simulink model "fft_test_BG"  (Simulink 7.4)
settings: Solver=ode45  StartTime=0.0  StopTime=5000  RelTol=1e-3
libraries: xbsIndex_r4 x4277, casper_library_misc x172, xbsReplacements_r4 x47, casper_library_delays x21, casper_library_reorder x4, casper_library_ffts_twiddle x2, simulink x2, xps_library x1
(part 23 of 37)

== system fft_test_BG/fft_BG_xblock/fft_biplex2/biplex_core/fft_stage_8/sync_delay ==
  In : Inport
  Constant : xbsIndex_r4/Constant  arith_type=Unsigned  const=1  n_bits=2  bin_pt=0  explicit_period=on  period=1  equ=P=C  opselect=C  inp2=PCIN>>17  opr=+  inp1=P  carry=CIN  dbl_ovrd=off  block_type=constant
      settings: block_version=11.4
  Constant1 : xbsIndex_r4/Constant  arith_type=Unsigned  const=0  n_bits=2  bin_pt=0  explicit_period=on  period=1  equ=P=C  opselect=C  inp2=PCIN>>17  opr=+  inp1=P  carry=CIN  dbl_ovrd=off  block_type=constant
      settings: block_version=11.4
  Constant2 : xbsIndex_r4/Constant  arith_type=Unsigned  const=1  n_bits=2  bin_pt=0  explicit_period=on  period=1  equ=P=C  opselect=C  inp2=PCIN>>17  opr=+  inp1=P  carry=CIN  dbl_ovrd=off  block_type=constant
      settings: block_version=11.4
  Constant3 : xbsIndex_r4/Constant  arith_type=Unsigned  const=1  n_bits=1  bin_pt=0  explicit_period=on  period=1  equ=P=C  opselect=C  inp2=PCIN>>17  opr=+  inp1=P  carry=CIN  dbl_ovrd=off  block_type=constant
      settings: block_version=11.4
  Counter : xbsIndex_r4/Counter  cnt_type=Free Running  cnt_to=Inf  operation=Down  start_count=0  cnt_by_val=1  arith_type=Unsigned  n_bits=2  bin_pt=0  load_pin=on  rst=off  en=on  explicit_period=on  period=1  dbl_ovrd=off  use_behavioral_HDL=off  implementation=Fabric  use_rpm=on  block_type=counter
      settings: block_version=11.4
  Logical : xbsIndex_r4/Logical  logical_function=OR  inputs=2  en=off  latency=0  precision=Full  arith_type=Unsigned  n_bits=8  bin_pt=2  align_bp=on  dbl_ovrd=off  block_type=logical
      settings: block_version=11.4
  Mux : xbsIndex_r4/Mux  inputs=2  en=off  latency=0  precision=Full  arith_type="Signed  (2's comp)"  n_bits=8  bin_pt=2  quantization=Truncate  overflow=Wrap  dbl_ovrd=off  block_type=mux
      settings: block_version=11.4
  Relational : xbsIndex_r4/Relational  mode=a=b  en=off  latency=0  dbl_ovrd=off  block_type=relational
  Relational1 : xbsIndex_r4/Relational  mode=a!=b  en=off  latency=0  dbl_ovrd=off  block_type=relational
  warn period Logical0 : xbsReplacements_r4/Warning  SystemSampleTime=-1  period=1
  warn period Relational10 : xbsReplacements_r4/Warning  SystemSampleTime=-1  period=1
  Out : Outport
  wires:
  In/1 -> Mux/2, Logical/1, Counter/1
  Counter/1 -> Relational1/1, Relational/2
  Relational1/1 -> Logical/2
  Constant1/1 -> Relational1/2
  Relational/1 -> Mux/3
  Constant/1 -> Relational/1
  Mux/1 -> Out/1
  Constant3/1 -> Mux/1
  Logical/1 -> Counter/3
  Constant2/1 -> Counter/2

== system fft_test_BG/fft_BG_xblock/fft_biplex2/biplex_cplx_unscrambler/reorder/sync_delay_en ==
  In : Inport
  en : Inport  Port=2
  Constant : xbsIndex_r4/Constant  arith_type=Unsigned  const=1  n_bits=9  bin_pt=0  explicit_period=on  period=1  equ=P=C  opselect=C  inp2=PCIN>>17  opr=+  inp1=P  carry=CIN  dbl_ovrd=off  block_type=constant
      settings: block_version=11.4
  Constant1 : xbsIndex_r4/Constant  arith_type=Unsigned  const=0  n_bits=9  bin_pt=0  explicit_period=on  period=1  equ=P=C  opselect=C  inp2=PCIN>>17  opr=+  inp1=P  carry=CIN  dbl_ovrd=off  block_type=constant
      settings: block_version=11.4
  Constant2 : xbsIndex_r4/Constant  arith_type=Unsigned  const=256  n_bits=9  bin_pt=0  explicit_period=on  period=1  equ=P=C  opselect=C  inp2=PCIN>>17  opr=+  inp1=P  carry=CIN  dbl_ovrd=off  block_type=constant
      settings: block_version=11.4
  Constant3 : xbsIndex_r4/Constant  arith_type=Unsigned  const=1  n_bits=1  bin_pt=0  explicit_period=on  period=1  equ=P=C  opselect=C  inp2=PCIN>>17  opr=+  inp1=P  carry=CIN  dbl_ovrd=off  block_type=constant
      settings: block_version=11.4
  Counter : xbsIndex_r4/Counter  cnt_type=Free Running  cnt_to=Inf  operation=Down  start_count=0  cnt_by_val=1  arith_type=Unsigned  n_bits=9  bin_pt=0  load_pin=on  rst=off  en=on  explicit_period=on  period=1  dbl_ovrd=off  use_behavioral_HDL=off  implementation=Fabric  use_rpm=on  block_type=counter
      settings: block_version=11.4
  Logical : xbsIndex_r4/Logical  logical_function=OR  inputs=2  en=off  latency=0  precision=Full  arith_type=Unsigned  n_bits=8  bin_pt=2  align_bp=on  dbl_ovrd=off  block_type=logical
      settings: block_version=11.4
  Logical1 : xbsIndex_r4/Logical  logical_function=AND  inputs=2  en=off  latency=0  precision=Full  arith_type=Unsigned  n_bits=8  bin_pt=2  align_bp=on  dbl_ovrd=off  block_type=logical
      settings: block_version=11.4
  Mux : xbsIndex_r4/Mux  inputs=2  en=off  latency=0  precision=Full  arith_type="Signed  (2's comp)"  n_bits=8  bin_pt=2  quantization=Truncate  overflow=Wrap  dbl_ovrd=off  block_type=mux
      settings: block_version=11.4
  Relational : xbsIndex_r4/Relational  mode=a=b  en=off  latency=0  dbl_ovrd=off  block_type=relational
  Relational1 : xbsIndex_r4/Relational  mode=a!=b  en=off  latency=0  dbl_ovrd=off  block_type=relational
  warn period Logical0 : xbsReplacements_r4/Warning  SystemSampleTime=-1  period=1
  warn period Logical10 : xbsReplacements_r4/Warning  SystemSampleTime=-1  period=1
  warn period Relational10 : xbsReplacements_r4/Warning  SystemSampleTime=-1  period=1
  Out : Outport
  wires:
  In/1 -> Mux/2, Logical/1, Counter/1
  Counter/1 -> Relational1/1, Relational/2
  Relational1/1 -> Logical/2
  Constant1/1 -> Relational1/2
  Relational/1 -> Mux/3
  Constant/1 -> Relational/1
  Mux/1 -> Out/1
  Constant3/1 -> Mux/1
  Logical1/1 -> Counter/3
  en/1 -> Logical1/2
  Logical/1 -> Logical1/1
  Constant2/1 -> Counter/2

== system fft_test_BG/fft_BG_xblock/fft_biplex2/biplex_cplx_unscrambler/reorder1/sync_delay_en ==
  In : Inport
  en : Inport  Port=2
  Constant : xbsIndex_r4/Constant  arith_type=Unsigned  const=1  n_bits=9  bin_pt=0  explicit_period=on  period=1  equ=P=C  opselect=C  inp2=PCIN>>17  opr=+  inp1=P  carry=CIN  dbl_ovrd=off  block_type=constant
      settings: block_version=11.4
  Constant1 : xbsIndex_r4/Constant  arith_type=Unsigned  const=0  n_bits=9  bin_pt=0  explicit_period=on  period=1  equ=P=C  opselect=C  inp2=PCIN>>17  opr=+  inp1=P  carry=CIN  dbl_ovrd=off  block_type=constant
      settings: block_version=11.4
  Constant2 : xbsIndex_r4/Constant  arith_type=Unsigned  const=256  n_bits=9  bin_pt=0  explicit_period=on  period=1  equ=P=C  opselect=C  inp2=PCIN>>17  opr=+  inp1=P  carry=CIN  dbl_ovrd=off  block_type=constant
      settings: block_version=11.4
  Constant3 : xbsIndex_r4/Constant  arith_type=Unsigned  const=1  n_bits=1  bin_pt=0  explicit_period=on  period=1  equ=P=C  opselect=C  inp2=PCIN>>17  opr=+  inp1=P  carry=CIN  dbl_ovrd=off  block_type=constant
      settings: block_version=11.4
  Counter : xbsIndex_r4/Counter  cnt_type=Free Running  cnt_to=Inf  operation=Down  start_count=0  cnt_by_val=1  arith_type=Unsigned  n_bits=9  bin_pt=0  load_pin=on  rst=off  en=on  explicit_period=on  period=1  dbl_ovrd=off  use_behavioral_HDL=off  implementation=Fabric  use_rpm=on  block_type=counter
      settings: block_version=11.4
  Logical : xbsIndex_r4/Logical  logical_function=OR  inputs=2  en=off  latency=0  precision=Full  arith_type=Unsigned  n_bits=8  bin_pt=2  align_bp=on  dbl_ovrd=off  block_type=logical
      settings: block_version=11.4
  Logical1 : xbsIndex_r4/Logical  logical_function=AND  inputs=2  en=off  latency=0  precision=Full  arith_type=Unsigned  n_bits=8  bin_pt=2  align_bp=on  dbl_ovrd=off  block_type=logical
      settings: block_version=11.4
  Mux : xbsIndex_r4/Mux  inputs=2  en=off  latency=0  precision=Full  arith_type="Signed  (2's comp)"  n_bits=8  bin_pt=2  quantization=Truncate  overflow=Wrap  dbl_ovrd=off  block_type=mux
      settings: block_version=11.4
  Relational : xbsIndex_r4/Relational  mode=a=b  en=off  latency=0  dbl_ovrd=off  block_type=relational
  Relational1 : xbsIndex_r4/Relational  mode=a!=b  en=off  latency=0  dbl_ovrd=off  block_type=relational
  warn period Logical0 : xbsReplacements_r4/Warning  SystemSampleTime=-1  period=1
  warn period Logical10 : xbsReplacements_r4/Warning  SystemSampleTime=-1  period=1
  warn period Relational10 : xbsReplacements_r4/Warning  SystemSampleTime=-1  period=1
  Out : Outport
  wires:
  In/1 -> Mux/2, Logical/1, Counter/1
  Counter/1 -> Relational1/1, Relational/2
  Relational1/1 -> Logical/2
  Constant1/1 -> Relational1/2
  Relational/1 -> Mux/3
  Constant/1 -> Relational/1
  Mux/1 -> Out/1
  Constant3/1 -> Mux/1
  Logical1/1 -> Counter/3
  en/1 -> Logical1/2
  Logical/1 -> Logical1/1
  Constant2/1 -> Counter/2

== system fft_test_BG/fft_BG_xblock/fft_direct_xblock/butterfly1_0/twiddle/c_to_ri_a ==
  c : Inport
  force_im : xbsIndex_r4/Reinterpret  force_arith_type=on  arith_type="Signed  (2's comp)"  force_bin_pt=on  bin_pt=16  block_type=reinterpret
      settings: infoedit="Changes signal type without altering the binary representation.   You can changed the signal between signed and unsigned, and relocate the binary point.<br><br>Hardware notes: In hardware this block costs nothing.<br><br>Example:  Suppose the input is 6 bits wide, signed, with 2 fractional bits, and the output is forced to unsigned with 0 fractional bits.  Then an input of -2.0 (1110.00 in binary 2's complement) becomes an output of 56 (111000 in binary)."
  force_re : xbsIndex_r4/Reinterpret  force_arith_type=on  arith_type="Signed  (2's comp)"  force_bin_pt=on  bin_pt=16  block_type=reinterpret
      settings: infoedit="Changes signal type without altering the binary representation.   You can changed the signal between signed and unsigned, and relocate the binary point.<br><br>Hardware notes: In hardware this block costs nothing.<br><br>Example:  Suppose the input is 6 bits wide, signed, with 2 fractional bits, and the output is forced to unsigned with 0 fractional bits.  Then an input of -2.0 (1110.00 in binary 2's complement) becomes an output of 56 (111000 in binary)."
  slice_im : xbsIndex_r4/Slice  nbits=17  boolean_output=off  mode=Lower Bit Location + Width  bit1=0  base1=MSB of Input  bit0=0  base0=LSB of Input  dbl_ovrd=off  block_type=slice
  slice_re : xbsIndex_r4/Slice  nbits=17  boolean_output=off  mode=Upper Bit Location + Width  bit1=0  base1=MSB of Input  bit0=0  base0=LSB of Input  dbl_ovrd=off  block_type=slice
  re : Outport
  im : Outport  Port=2
  wires:
  c/1 -> slice_re/1, slice_im/1
  slice_re/1 -> force_re/1
  slice_im/1 -> force_im/1
  force_re/1 -> re/1
  force_im/1 -> im/1

== system fft_test_BG/fft_BG_xblock/fft_direct_xblock/butterfly1_0/twiddle/c_to_ri_b ==
  c : Inport
  force_im : xbsIndex_r4/Reinterpret  force_arith_type=on  arith_type="Signed  (2's comp)"  force_bin_pt=on  bin_pt=16  block_type=reinterpret
      settings: infoedit="Changes signal type without altering the binary representation.   You can changed the signal between signed and unsigned, and relocate the binary point.<br><br>Hardware notes: In hardware this block costs nothing.<br><br>Example:  Suppose the input is 6 bits wide, signed, with 2 fractional bits, and the output is forced to unsigned with 0 fractional bits.  Then an input of -2.0 (1110.00 in binary 2's complement) becomes an output of 56 (111000 in binary)."
  force_re : xbsIndex_r4/Reinterpret  force_arith_type=on  arith_type="Signed  (2's comp)"  force_bin_pt=on  bin_pt=16  block_type=reinterpret
      settings: infoedit="Changes signal type without altering the binary representation.   You can changed the signal between signed and unsigned, and relocate the binary point.<br><br>Hardware notes: In hardware this block costs nothing.<br><br>Example:  Suppose the input is 6 bits wide, signed, with 2 fractional bits, and the output is forced to unsigned with 0 fractional bits.  Then an input of -2.0 (1110.00 in binary 2's complement) becomes an output of 56 (111000 in binary)."
  slice_im : xbsIndex_r4/Slice  nbits=17  boolean_output=off  mode=Lower Bit Location + Width  bit1=0  base1=MSB of Input  bit0=0  base0=LSB of Input  dbl_ovrd=off  block_type=slice
  slice_re : xbsIndex_r4/Slice  nbits=17  boolean_output=off  mode=Upper Bit Location + Width  bit1=0  base1=MSB of Input  bit0=0  base0=LSB of Input  dbl_ovrd=off  block_type=slice
  re : Outport
  im : Outport  Port=2
  wires:
  c/1 -> slice_re/1, slice_im/1
  slice_re/1 -> force_re/1
  slice_im/1 -> force_im/1
  force_re/1 -> re/1
  force_im/1 -> im/1

== system fft_test_BG/fft_BG_xblock/fft_direct_xblock/butterfly1_0/twiddle/c_to_ri_w ==
  c : Inport
  force_im : xbsIndex_r4/Reinterpret  force_arith_type=on  arith_type="Signed  (2's comp)"  force_bin_pt=on  bin_pt=15  block_type=reinterpret
      settings: infoedit="Changes signal type without altering the binary representation.   You can changed the signal between signed and unsigned, and relocate the binary point.<br><br>Hardware notes: In hardware this block costs nothing.<br><br>Example:  Suppose the input is 6 bits wide, signed, with 2 fractional bits, and the output is forced to unsigned with 0 fractional bits.  Then an input of -2.0 (1110.00 in binary 2's complement) becomes an output of 56 (111000 in binary)."
  force_re : xbsIndex_r4/Reinterpret  force_arith_type=on  arith_type="Signed  (2's comp)"  force_bin_pt=on  bin_pt=15  block_type=reinterpret
      settings: infoedit="Changes signal type without altering the binary representation.   You can changed the signal between signed and unsigned, and relocate the binary point.<br><br>Hardware notes: In hardware this block costs nothing.<br><br>Example:  Suppose the input is 6 bits wide, signed, with 2 fractional bits, and the output is forced to unsigned with 0 fractional bits.  Then an input of -2.0 (1110.00 in binary 2's complement) becomes an output of 56 (111000 in binary)."
  slice_im : xbsIndex_r4/Slice  nbits=17  boolean_output=off  mode=Lower Bit Location + Width  bit1=0  base1=MSB of Input  bit0=0  base0=LSB of Input  dbl_ovrd=off  block_type=slice
  slice_re : xbsIndex_r4/Slice  nbits=17  boolean_output=off  mode=Upper Bit Location + Width  bit1=0  base1=MSB of Input  bit0=0  base0=LSB of Input  dbl_ovrd=off  block_type=slice
  re : Outport
  im : Outport  Port=2
  wires:
  c/1 -> slice_re/1, slice_im/1
  slice_re/1 -> force_re/1
  slice_im/1 -> force_im/1
  force_re/1 -> re/1
  force_im/1 -> im/1

== system fft_test_BG/fft_BG_xblock/fft_direct_xblock/butterfly1_0/twiddle/coeff_gen ==
  rst : Inport
  Counter : xbsIndex_r4/Counter  cnt_type=Free Running  cnt_to=Inf  operation=Up  start_count=0  cnt_by_val=1  arith_type=Unsigned  n_bits=8  bin_pt=0  load_pin=off  rst=on  en=off  explicit_period=on  period=1  dbl_ovrd=off  use_behavioral_HDL=off  implementation=Fabric  block_type=counter
      settings: block_version=11.4
  ROM : xbsIndex_r4/ROM  depth=256  initVector=[1 0.99993896484375 0.99969482421875 0.99932861328125 0.998809814453125 0.99810791015625 0.997283935546875 0.996307373046875 0.99517822265625 0.993896484375 0.99249267578125 0.99090576171875 0.989166259765625 0.9873046875 0.98529052734375 0.98309326171875 0.98077392578125 0.97833251953125 0.9757080078125 0.972930908203125 0.97003173828125 0.96697998046875 0.963775634765625 0.960418701171875 0.956939697265625 0.95330810546875 0.94952392578125 0.94561767578125 0.941558837890625 0.937347412109375 0.9329833984375 0.928497314453125 0.92388916015625 0.91912841796875 0.914215087890625 0.9091796875 0.90399169921875 0.898681640625 0.893218994140625 0.88763427734375 0.881927490234375 0.876068115234375 0.870086669921875 0.863983154296875 0.85772705078125 0.851348876953125 0.8448486328125 0.838226318359375 0.83148193359375 0.8245849609375 0.817596435546875 0.810455322265625 0.80322265625 0.79583740234375 0.788360595703125 0.780731201171875 0.77301025390625 0.765167236328125 0.7572021484375 0.7491455078125 0.740936279296875 0.732666015625 0.7242431640625 0.715728759765625 0.70709228515625 0.6983642578125 0.689544677734375 0.68060302734375 0.67156982421875 0.66241455078125 0.653167724609375 0.643829345703125 0.6343994140625 0.624847412109375 0.615234375 0.605499267578125 0.595703125 0.585784912109375 0.5758056640625 0.56573486328125 0.555572509765625 0.545318603515625 0.535003662109375 0.52459716796875 0.51409912109375 0.5035400390625 0.492889404296875 0.482177734375 0.471405029296875 0.460540771484375 0.449615478515625 0.438629150390625 0.42755126953125 0.41644287109375 0.405242919921875 0.39398193359375 0.3826904296875 0.371307373046875 0.359893798828125 0.348419189453125 0.336883544921875 0.3253173828125 0.313690185546875 0.302001953125 0.290283203125 0.278533935546875 0.2667236328125 0.254852294921875 0.24298095703125 0.231048583984375 0.2191162109375 0.207122802734375 0.195098876953125 0.18304443359375 0.17095947265625 0.158843994140625 0.146728515625 0.13458251953125 0.122406005859375 0.1102294921875 0.0980224609375 0.085784912109375 0.073577880859375 0.061309814453125 0.049072265625 0.03680419921875 0.0245361328125 0.01226806640625 0 -0.01226806640625 -0.0245361328125 -0.03680419921875 -0.049072265625 -0.061309814453125 -0.073577880859375 -0.085784912109375 -0.0980224609375 -0.1102294921875 -0.122406005859375 -0.13458251953125 -0.146728515625 -0.158843994140625 -0.17095947265625 -0.18304443359375 -0.195098876953125 -0.207122802734375 -0.2191162109375 -0.231048583984375 -0.24298095703125 -0.254852294921875 -0.2667236328125 -0.278533935546875 -0.290283203125 -0.302001953125 -0.313690185546875 -0.3253173828125 -0.336883544921875 -0.348419189453125 -0.359893798828125 -0.371307373046875 -0.3826904296875 -0.39398193359375 -0.405242919921875 -0.41644287109375 -0.42755126953125 -0.438629150390625 -0.449615478515625 -0.460540771484375 -0.471405029296875 -0.482177734375 -0.492889404296875 -0.5035400390625 -0.51409912109375 -0.52459716796875 -0.535003662109375 -0.545318603515625 -0.555572509765625 -0.56573486328125 -0.5758056640625 -0.585784912109375 -0.595703125 -0.605499267578125 -0.615234375 -0.624847412109375 -0.6343994140625 -0.643829345703125 -0.653167724609375 -0.66241455078125 -0.67156982421875 -0.68060302734375 -0.689544677734375 -0.6983642578125 -0.70709228515625 -0.715728759765625 -0.7242431640625 -0.732666015625 -0.740936279296875 -0.7491455078125 -0.7572021484375 -0.765167236328125 -0.77301025390625 -0.780731201171875 -0.788360595703125 -0.79583740234375 -0.80322265625 -0.810455322265625 -0.817596435546875 -0.8245849609375 -0.83148193359375 -0.838226318359375 -0.8448486328125 -0.851348876953125 -0.85772705078125 -0.863983154296875 -0.870086669921875 -0.876068115234375 -0.881927490234375 -0.88763427734375 -0.893218994140625 -0.898681640625 -0.90399169921875 -0.9091796875 -0.914215087890625 -0.91912841796875 -0.92388916015625 -0.928497314453125 -0.9329833984375 -0.937347412109375 -0.941558837890625 -0.94561767578125 ...(+469 chars)]  distributed_mem=Block RAM  rst=off  init_reg=0  en=off  latency=2  arith_type="Signed  (2's comp)"  n_bits=17  bin_pt=15  dbl_ovrd=off  optimize=Area  use_rpm=on  block_type=sprom
      settings: block_version=11.4
  ROM1 : xbsIndex_r4/ROM  depth=256  initVector=[0 -0.01226806640625 -0.0245361328125 -0.03680419921875 -0.049072265625 -0.061309814453125 -0.073577880859375 -0.085784912109375 -0.0980224609375 -0.1102294921875 -0.122406005859375 -0.13458251953125 -0.146728515625 -0.158843994140625 -0.17095947265625 -0.18304443359375 -0.195098876953125 -0.207122802734375 -0.2191162109375 -0.231048583984375 -0.24298095703125 -0.254852294921875 -0.2667236328125 -0.278533935546875 -0.290283203125 -0.302001953125 -0.313690185546875 -0.3253173828125 -0.336883544921875 -0.348419189453125 -0.359893798828125 -0.371307373046875 -0.3826904296875 -0.39398193359375 -0.405242919921875 -0.41644287109375 -0.42755126953125 -0.438629150390625 -0.449615478515625 -0.460540771484375 -0.471405029296875 -0.482177734375 -0.492889404296875 -0.5035400390625 -0.51409912109375 -0.52459716796875 -0.535003662109375 -0.545318603515625 -0.555572509765625 -0.56573486328125 -0.5758056640625 -0.585784912109375 -0.595703125 -0.605499267578125 -0.615234375 -0.624847412109375 -0.6343994140625 -0.643829345703125 -0.653167724609375 -0.66241455078125 -0.67156982421875 -0.68060302734375 -0.689544677734375 -0.6983642578125 -0.70709228515625 -0.715728759765625 -0.7242431640625 -0.732666015625 -0.740936279296875 -0.7491455078125 -0.7572021484375 -0.765167236328125 -0.77301025390625 -0.780731201171875 -0.788360595703125 -0.79583740234375 -0.80322265625 -0.810455322265625 -0.817596435546875 -0.8245849609375 -0.83148193359375 -0.838226318359375 -0.8448486328125 -0.851348876953125 -0.85772705078125 -0.863983154296875 -0.870086669921875 -0.876068115234375 -0.881927490234375 -0.88763427734375 -0.893218994140625 -0.898681640625 -0.90399169921875 -0.9091796875 -0.914215087890625 -0.91912841796875 -0.92388916015625 -0.928497314453125 -0.9329833984375 -0.937347412109375 -0.941558837890625 -0.94561767578125 -0.94952392578125 -0.95330810546875 -0.956939697265625 -0.960418701171875 -0.963775634765625 -0.96697998046875 -0.97003173828125 -0.972930908203125 -0.9757080078125 -0.97833251953125 -0.98077392578125 -0.98309326171875 -0.98529052734375 -0.9873046875 -0.989166259765625 -0.99090576171875 -0.99249267578125 -0.993896484375 -0.99517822265625 -0.996307373046875 -0.997283935546875 -0.99810791015625 -0.998809814453125 -0.99932861328125 -0.99969482421875 -0.99993896484375 -1 -0.99993896484375 -0.99969482421875 -0.99932861328125 -0.998809814453125 -0.99810791015625 -0.997283935546875 -0.996307373046875 -0.99517822265625 -0.993896484375 -0.99249267578125 -0.99090576171875 -0.989166259765625 -0.9873046875 -0.98529052734375 -0.98309326171875 -0.98077392578125 -0.97833251953125 -0.9757080078125 -0.972930908203125 -0.97003173828125 -0.96697998046875 -0.963775634765625 -0.960418701171875 -0.956939697265625 -0.95330810546875 -0.94952392578125 -0.94561767578125 -0.941558837890625 -0.937347412109375 -0.9329833984375 -0.928497314453125 -0.92388916015625 -0.91912841796875 -0.914215087890625 -0.9091796875 -0.90399169921875 -0.898681640625 -0.893218994140625 -0.88763427734375 -0.881927490234375 -0.876068115234375 -0.870086669921875 -0.863983154296875 -0.85772705078125 -0.851348876953125 -0.8448486328125 -0.838226318359375 -0.83148193359375 -0.8245849609375 -0.817596435546875 -0.810455322265625 -0.80322265625 -0.79583740234375 -0.788360595703125 -0.780731201171875 -0.77301025390625 -0.765167236328125 -0.7572021484375 -0.7491455078125 -0.740936279296875 -0.732666015625 -0.7242431640625 -0.715728759765625 -0.70709228515625 -0.6983642578125 -0.689544677734375 -0.68060302734375 -0.67156982421875 -0.66241455078125 -0.653167724609375 -0.643829345703125 -0.6343994140625 -0.624847412109375 -0.615234375 -0.605499267578125 -0.595703125 -0.585784912109375 -0.5758056640625 -0.56573486328125 -0.555572509765625 -0.545318603515625 -0.535003662109375 -0.52459716796875 -0.51409912109375 -0.5035400390625 -0.492889404296875 -0.482177734375 -0.471405029296875 -0.460540771484375 -0.449615478515625 -0.438629150390625 -0.42755126953125 -0.41644287109375 -0.405242919921875 ...(+594 chars)]  distributed_mem=Block RAM  rst=off  init_reg=0  en=off  latency=2  arith_type="Signed  (2's comp)"  n_bits=17  bin_pt=15  dbl_ovrd=off  optimize=Area  use_rpm=on  block_type=sprom
      settings: block_version=11.4
  Slice : xbsIndex_r4/Slice  nbits=8  boolean_output=off  mode=Upper Bit Location + Width  bit1=0  base1=MSB of Input  bit0=0  base0=LSB of Input  dbl_ovrd=off  block_type=slice
  ri_to_c : casper_library_misc/ri_to_c  SystemSampleTime=-1
  w : Outport
  wires:
  Slice/1 -> ROM1/1, ROM/1
  ri_to_c/1 -> w/1
  ROM1/1 -> ri_to_c/2
  ROM/1 -> ri_to_c/1
  Counter/1 -> Slice/1
  rst/1 -> Counter/1

== system fft_test_BG/fft_BG_xblock/fft_direct_xblock/butterfly1_0/twiddle/mults ==
  a1 : Inport
  b1 : Inport  Port=2
  a2 : Inport  Port=3
  b2 : Inport  Port=4
  a3 : Inport  Port=5
  b3 : Inport  Port=6
  a4 : Inport  Port=7
  b4 : Inport  Port=8
  mult_{1..4} (4 alike) : xbsIndex_r4/Mult  precision=Full  arith_type="Signed  (2's comp)"  n_bits=16  bin_pt=14  quantization="Round  (unbiased: +/- Inf)"  overflow=Saturate  en=off  latency=2  dbl_ovrd=off  use_behavioral_HDL=off  opt=Speed  use_embedded=on  optimum_pipeline=off  pipeline=on  use_rpm=on  placement_style=Triangular  block_type=mult
      settings: block_version=11.4
  c1 : Outport
  c2 : Outport  Port=2
  c3 : Outport  Port=3
  c4 : Outport  Port=4
  wires:
  mult_4/1 -> c4/1
  b4/1 -> mult_4/2
  a4/1 -> mult_4/1
  mult_3/1 -> c3/1
  b3/1 -> mult_3/2
  a3/1 -> mult_3/1
  mult_2/1 -> c2/1
  b2/1 -> mult_2/2
  a2/1 -> mult_2/1
  mult_1/1 -> c1/1
  b1/1 -> mult_1/2
  a1/1 -> mult_1/1

== system fft_test_BG/fft_BG_xblock/fft_direct_xblock/butterfly1_1/twiddle/c_to_ri_a ==
  c : Inport
  force_im : xbsIndex_r4/Reinterpret  force_arith_type=on  arith_type="Signed  (2's comp)"  force_bin_pt=on  bin_pt=16  block_type=reinterpret
      settings: infoedit="Changes signal type without altering the binary representation.   You can changed the signal between signed and unsigned, and relocate the binary point.<br><br>Hardware notes: In hardware this block costs nothing.<br><br>Example:  Suppose the input is 6 bits wide, signed, with 2 fractional bits, and the output is forced to unsigned with 0 fractional bits.  Then an input of -2.0 (1110.00 in binary 2's complement) becomes an output of 56 (111000 in binary)."
  force_re : xbsIndex_r4/Reinterpret  force_arith_type=on  arith_type="Signed  (2's comp)"  force_bin_pt=on  bin_pt=16  block_type=reinterpret
      settings: infoedit="Changes signal type without altering the binary representation.   You can changed the signal between signed and unsigned, and relocate the binary point.<br><br>Hardware notes: In hardware this block costs nothing.<br><br>Example:  Suppose the input is 6 bits wide, signed, with 2 fractional bits, and the output is forced to unsigned with 0 fractional bits.  Then an input of -2.0 (1110.00 in binary 2's complement) becomes an output of 56 (111000 in binary)."
  slice_im : xbsIndex_r4/Slice  nbits=17  boolean_output=off  mode=Lower Bit Location + Width  bit1=0  base1=MSB of Input  bit0=0  base0=LSB of Input  dbl_ovrd=off  block_type=slice
  slice_re : xbsIndex_r4/Slice  nbits=17  boolean_output=off  mode=Upper Bit Location + Width  bit1=0  base1=MSB of Input  bit0=0  base0=LSB of Input  dbl_ovrd=off  block_type=slice
  re : Outport
  im : Outport  Port=2
  wires:
  c/1 -> slice_re/1, slice_im/1
  slice_re/1 -> force_re/1
  slice_im/1 -> force_im/1
  force_re/1 -> re/1
  force_im/1 -> im/1

== system fft_test_BG/fft_BG_xblock/fft_direct_xblock/butterfly1_1/twiddle/c_to_ri_b ==
  c : Inport
  force_im : xbsIndex_r4/Reinterpret  force_arith_type=on  arith_type="Signed  (2's comp)"  force_bin_pt=on  bin_pt=16  block_type=reinterpret
      settings: infoedit="Changes signal type without altering the binary representation.   You can changed the signal between signed and unsigned, and relocate the binary point.<br><br>Hardware notes: In hardware this block costs nothing.<br><br>Example:  Suppose the input is 6 bits wide, signed, with 2 fractional bits, and the output is forced to unsigned with 0 fractional bits.  Then an input of -2.0 (1110.00 in binary 2's complement) becomes an output of 56 (111000 in binary)."
  force_re : xbsIndex_r4/Reinterpret  force_arith_type=on  arith_type="Signed  (2's comp)"  force_bin_pt=on  bin_pt=16  block_type=reinterpret
      settings: infoedit="Changes signal type without altering the binary representation.   You can changed the signal between signed and unsigned, and relocate the binary point.<br><br>Hardware notes: In hardware this block costs nothing.<br><br>Example:  Suppose the input is 6 bits wide, signed, with 2 fractional bits, and the output is forced to unsigned with 0 fractional bits.  Then an input of -2.0 (1110.00 in binary 2's complement) becomes an output of 56 (111000 in binary)."
  slice_im : xbsIndex_r4/Slice  nbits=17  boolean_output=off  mode=Lower Bit Location + Width  bit1=0  base1=MSB of Input  bit0=0  base0=LSB of Input  dbl_ovrd=off  block_type=slice
  slice_re : xbsIndex_r4/Slice  nbits=17  boolean_output=off  mode=Upper Bit Location + Width  bit1=0  base1=MSB of Input  bit0=0  base0=LSB of Input  dbl_ovrd=off  block_type=slice
  re : Outport
  im : Outport  Port=2
  wires:
  c/1 -> slice_re/1, slice_im/1
  slice_re/1 -> force_re/1
  slice_im/1 -> force_im/1
  force_re/1 -> re/1
  force_im/1 -> im/1

== system fft_test_BG/fft_BG_xblock/fft_direct_xblock/butterfly1_1/twiddle/c_to_ri_w ==
  c : Inport
  force_im : xbsIndex_r4/Reinterpret  force_arith_type=on  arith_type="Signed  (2's comp)"  force_bin_pt=on  bin_pt=15  block_type=reinterpret
      settings: infoedit="Changes signal type without altering the binary representation.   You can changed the signal between signed and unsigned, and relocate the binary point.<br><br>Hardware notes: In hardware this block costs nothing.<br><br>Example:  Suppose the input is 6 bits wide, signed, with 2 fractional bits, and the output is forced to unsigned with 0 fractional bits.  Then an input of -2.0 (1110.00 in binary 2's complement) becomes an output of 56 (111000 in binary)."
  force_re : xbsIndex_r4/Reinterpret  force_arith_type=on  arith_type="Signed  (2's comp)"  force_bin_pt=on  bin_pt=15  block_type=reinterpret
      settings: infoedit="Changes signal type without altering the binary representation.   You can changed the signal between signed and unsigned, and relocate the binary point.<br><br>Hardware notes: In hardware this block costs nothing.<br><br>Example:  Suppose the input is 6 bits wide, signed, with 2 fractional bits, and the output is forced to unsigned with 0 fractional bits.  Then an input of -2.0 (1110.00 in binary 2's complement) becomes an output of 56 (111000 in binary)."
  slice_im : xbsIndex_r4/Slice  nbits=17  boolean_output=off  mode=Lower Bit Location + Width  bit1=0  base1=MSB of Input  bit0=0  base0=LSB of Input  dbl_ovrd=off  block_type=slice
  slice_re : xbsIndex_r4/Slice  nbits=17  boolean_output=off  mode=Upper Bit Location + Width  bit1=0  base1=MSB of Input  bit0=0  base0=LSB of Input  dbl_ovrd=off  block_type=slice
  re : Outport
  im : Outport  Port=2
  wires:
  c/1 -> slice_re/1, slice_im/1
  slice_re/1 -> force_re/1
  slice_im/1 -> force_im/1
  force_re/1 -> re/1
  force_im/1 -> im/1

== system fft_test_BG/fft_BG_xblock/fft_direct_xblock/butterfly1_1/twiddle/coeff_gen ==
  rst : Inport
  Counter : xbsIndex_r4/Counter  cnt_type=Free Running  cnt_to=Inf  operation=Up  start_count=0  cnt_by_val=1  arith_type=Unsigned  n_bits=8  bin_pt=0  load_pin=off  rst=on  en=off  explicit_period=on  period=1  dbl_ovrd=off  use_behavioral_HDL=off  implementation=Fabric  block_type=counter
      settings: block_version=11.4
  ROM : xbsIndex_r4/ROM  depth=256  initVector=[1 0.99993896484375 0.99969482421875 0.99932861328125 0.998809814453125 0.99810791015625 0.997283935546875 0.996307373046875 0.99517822265625 0.993896484375 0.99249267578125 0.99090576171875 0.989166259765625 0.9873046875 0.98529052734375 0.98309326171875 0.98077392578125 0.97833251953125 0.9757080078125 0.972930908203125 0.97003173828125 0.96697998046875 0.963775634765625 0.960418701171875 0.956939697265625 0.95330810546875 0.94952392578125 0.94561767578125 0.941558837890625 0.937347412109375 0.9329833984375 0.928497314453125 0.92388916015625 0.91912841796875 0.914215087890625 0.9091796875 0.90399169921875 0.898681640625 0.893218994140625 0.88763427734375 0.881927490234375 0.876068115234375 0.870086669921875 0.863983154296875 0.85772705078125 0.851348876953125 0.8448486328125 0.838226318359375 0.83148193359375 0.8245849609375 0.817596435546875 0.810455322265625 0.80322265625 0.79583740234375 0.788360595703125 0.780731201171875 0.77301025390625 0.765167236328125 0.7572021484375 0.7491455078125 0.740936279296875 0.732666015625 0.7242431640625 0.715728759765625 0.70709228515625 0.6983642578125 0.689544677734375 0.68060302734375 0.67156982421875 0.66241455078125 0.653167724609375 0.643829345703125 0.6343994140625 0.624847412109375 0.615234375 0.605499267578125 0.595703125 0.585784912109375 0.5758056640625 0.56573486328125 0.555572509765625 0.545318603515625 0.535003662109375 0.52459716796875 0.51409912109375 0.5035400390625 0.492889404296875 0.482177734375 0.471405029296875 0.460540771484375 0.449615478515625 0.438629150390625 0.42755126953125 0.41644287109375 0.405242919921875 0.39398193359375 0.3826904296875 0.371307373046875 0.359893798828125 0.348419189453125 0.336883544921875 0.3253173828125 0.313690185546875 0.302001953125 0.290283203125 0.278533935546875 0.2667236328125 0.254852294921875 0.24298095703125 0.231048583984375 0.2191162109375 0.207122802734375 0.195098876953125 0.18304443359375 0.17095947265625 0.158843994140625 0.146728515625 0.13458251953125 0.122406005859375 0.1102294921875 0.0980224609375 0.085784912109375 0.073577880859375 0.061309814453125 0.049072265625 0.03680419921875 0.0245361328125 0.01226806640625 0 -0.01226806640625 -0.0245361328125 -0.03680419921875 -0.049072265625 -0.061309814453125 -0.073577880859375 -0.085784912109375 -0.0980224609375 -0.1102294921875 -0.122406005859375 -0.13458251953125 -0.146728515625 -0.158843994140625 -0.17095947265625 -0.18304443359375 -0.195098876953125 -0.207122802734375 -0.2191162109375 -0.231048583984375 -0.24298095703125 -0.254852294921875 -0.2667236328125 -0.278533935546875 -0.290283203125 -0.302001953125 -0.313690185546875 -0.3253173828125 -0.336883544921875 -0.348419189453125 -0.359893798828125 -0.371307373046875 -0.3826904296875 -0.39398193359375 -0.405242919921875 -0.41644287109375 -0.42755126953125 -0.438629150390625 -0.449615478515625 -0.460540771484375 -0.471405029296875 -0.482177734375 -0.492889404296875 -0.5035400390625 -0.51409912109375 -0.52459716796875 -0.535003662109375 -0.545318603515625 -0.555572509765625 -0.56573486328125 -0.5758056640625 -0.585784912109375 -0.595703125 -0.605499267578125 -0.615234375 -0.624847412109375 -0.6343994140625 -0.643829345703125 -0.653167724609375 -0.66241455078125 -0.67156982421875 -0.68060302734375 -0.689544677734375 -0.6983642578125 -0.70709228515625 -0.715728759765625 -0.7242431640625 -0.732666015625 -0.740936279296875 -0.7491455078125 -0.7572021484375 -0.765167236328125 -0.77301025390625 -0.780731201171875 -0.788360595703125 -0.79583740234375 -0.80322265625 -0.810455322265625 -0.817596435546875 -0.8245849609375 -0.83148193359375 -0.838226318359375 -0.8448486328125 -0.851348876953125 -0.85772705078125 -0.863983154296875 -0.870086669921875 -0.876068115234375 -0.881927490234375 -0.88763427734375 -0.893218994140625 -0.898681640625 -0.90399169921875 -0.9091796875 -0.914215087890625 -0.91912841796875 -0.92388916015625 -0.928497314453125 -0.9329833984375 -0.937347412109375 -0.941558837890625 -0.94561767578125 ...(+469 chars)]  distributed_mem=Block RAM  rst=off  init_reg=0  en=off  latency=2  arith_type="Signed  (2's comp)"  n_bits=17  bin_pt=15  dbl_ovrd=off  optimize=Area  use_rpm=on  block_type=sprom
      settings: block_version=11.4
  ROM1 : xbsIndex_r4/ROM  depth=256  initVector=[0 -0.01226806640625 -0.0245361328125 -0.03680419921875 -0.049072265625 -0.061309814453125 -0.073577880859375 -0.085784912109375 -0.0980224609375 -0.1102294921875 -0.122406005859375 -0.13458251953125 -0.146728515625 -0.158843994140625 -0.17095947265625 -0.18304443359375 -0.195098876953125 -0.207122802734375 -0.2191162109375 -0.231048583984375 -0.24298095703125 -0.254852294921875 -0.2667236328125 -0.278533935546875 -0.290283203125 -0.302001953125 -0.313690185546875 -0.3253173828125 -0.336883544921875 -0.348419189453125 -0.359893798828125 -0.371307373046875 -0.3826904296875 -0.39398193359375 -0.405242919921875 -0.41644287109375 -0.42755126953125 -0.438629150390625 -0.449615478515625 -0.460540771484375 -0.471405029296875 -0.482177734375 -0.492889404296875 -0.5035400390625 -0.51409912109375 -0.52459716796875 -0.535003662109375 -0.545318603515625 -0.555572509765625 -0.56573486328125 -0.5758056640625 -0.585784912109375 -0.595703125 -0.605499267578125 -0.615234375 -0.624847412109375 -0.6343994140625 -0.643829345703125 -0.653167724609375 -0.66241455078125 -0.67156982421875 -0.68060302734375 -0.689544677734375 -0.6983642578125 -0.70709228515625 -0.715728759765625 -0.7242431640625 -0.732666015625 -0.740936279296875 -0.7491455078125 -0.7572021484375 -0.765167236328125 -0.77301025390625 -0.780731201171875 -0.788360595703125 -0.79583740234375 -0.80322265625 -0.810455322265625 -0.817596435546875 -0.8245849609375 -0.83148193359375 -0.838226318359375 -0.8448486328125 -0.851348876953125 -0.85772705078125 -0.863983154296875 -0.870086669921875 -0.876068115234375 -0.881927490234375 -0.88763427734375 -0.893218994140625 -0.898681640625 -0.90399169921875 -0.9091796875 -0.914215087890625 -0.91912841796875 -0.92388916015625 -0.928497314453125 -0.9329833984375 -0.937347412109375 -0.941558837890625 -0.94561767578125 -0.94952392578125 -0.95330810546875 -0.956939697265625 -0.960418701171875 -0.963775634765625 -0.96697998046875 -0.97003173828125 -0.972930908203125 -0.9757080078125 -0.97833251953125 -0.98077392578125 -0.98309326171875 -0.98529052734375 -0.9873046875 -0.989166259765625 -0.99090576171875 -0.99249267578125 -0.993896484375 -0.99517822265625 -0.996307373046875 -0.997283935546875 -0.99810791015625 -0.998809814453125 -0.99932861328125 -0.99969482421875 -0.99993896484375 -1 -0.99993896484375 -0.99969482421875 -0.99932861328125 -0.998809814453125 -0.99810791015625 -0.997283935546875 -0.996307373046875 -0.99517822265625 -0.993896484375 -0.99249267578125 -0.99090576171875 -0.989166259765625 -0.9873046875 -0.98529052734375 -0.98309326171875 -0.98077392578125 -0.97833251953125 -0.9757080078125 -0.972930908203125 -0.97003173828125 -0.96697998046875 -0.963775634765625 -0.960418701171875 -0.956939697265625 -0.95330810546875 -0.94952392578125 -0.94561767578125 -0.941558837890625 -0.937347412109375 -0.9329833984375 -0.928497314453125 -0.92388916015625 -0.91912841796875 -0.914215087890625 -0.9091796875 -0.90399169921875 -0.898681640625 -0.893218994140625 -0.88763427734375 -0.881927490234375 -0.876068115234375 -0.870086669921875 -0.863983154296875 -0.85772705078125 -0.851348876953125 -0.8448486328125 -0.838226318359375 -0.83148193359375 -0.8245849609375 -0.817596435546875 -0.810455322265625 -0.80322265625 -0.79583740234375 -0.788360595703125 -0.780731201171875 -0.77301025390625 -0.765167236328125 -0.7572021484375 -0.7491455078125 -0.740936279296875 -0.732666015625 -0.7242431640625 -0.715728759765625 -0.70709228515625 -0.6983642578125 -0.689544677734375 -0.68060302734375 -0.67156982421875 -0.66241455078125 -0.653167724609375 -0.643829345703125 -0.6343994140625 -0.624847412109375 -0.615234375 -0.605499267578125 -0.595703125 -0.585784912109375 -0.5758056640625 -0.56573486328125 -0.555572509765625 -0.545318603515625 -0.535003662109375 -0.52459716796875 -0.51409912109375 -0.5035400390625 -0.492889404296875 -0.482177734375 -0.471405029296875 -0.460540771484375 -0.449615478515625 -0.438629150390625 -0.42755126953125 -0.41644287109375 -0.405242919921875 ...(+594 chars)]  distributed_mem=Block RAM  rst=off  init_reg=0  en=off  latency=2  arith_type="Signed  (2's comp)"  n_bits=17  bin_pt=15  dbl_ovrd=off  optimize=Area  use_rpm=on  block_type=sprom
      settings: block_version=11.4
  Slice : xbsIndex_r4/Slice  nbits=8  boolean_output=off  mode=Upper Bit Location + Width  bit1=0  base1=MSB of Input  bit0=0  base0=LSB of Input  dbl_ovrd=off  block_type=slice
  ri_to_c : casper_library_misc/ri_to_c  SystemSampleTime=-1
  w : Outport
  wires:
  Slice/1 -> ROM1/1, ROM/1
  ri_to_c/1 -> w/1
  ROM1/1 -> ri_to_c/2
  ROM/1 -> ri_to_c/1
  Counter/1 -> Slice/1
  rst/1 -> Counter/1

== system fft_test_BG/fft_BG_xblock/fft_direct_xblock/butterfly1_1/twiddle/mults ==
  a1 : Inport
  b1 : Inport  Port=2
  a2 : Inport  Port=3
  b2 : Inport  Port=4
  a3 : Inport  Port=5
  b3 : Inport  Port=6
  a4 : Inport  Port=7
  b4 : Inport  Port=8
  mult_{1..4} (4 alike) : xbsIndex_r4/Mult  precision=Full  arith_type="Signed  (2's comp)"  n_bits=16  bin_pt=14  quantization="Round  (unbiased: +/- Inf)"  overflow=Saturate  en=off  latency=2  dbl_ovrd=off  use_behavioral_HDL=off  opt=Speed  use_embedded=on  optimum_pipeline=off  pipeline=on  use_rpm=on  placement_style=Triangular  block_type=mult
      settings: block_version=11.4
  c1 : Outport
  c2 : Outport  Port=2
  c3 : Outport  Port=3
  c4 : Outport  Port=4
  wires:
  mult_4/1 -> c4/1
  b4/1 -> mult_4/2
  a4/1 -> mult_4/1
  mult_3/1 -> c3/1
  b3/1 -> mult_3/2
  a3/1 -> mult_3/1
  mult_2/1 -> c2/1
  b2/1 -> mult_2/2
  a2/1 -> mult_2/1
  mult_1/1 -> c1/1
  b1/1 -> mult_1/2
  a1/1 -> mult_1/1

== system fft_test_BG/fft_BG_xblock/fft_direct_xblock/butterfly2_0/twiddle/c_to_ri_a ==
  c : Inport
  force_im : xbsIndex_r4/Reinterpret  force_arith_type=on  arith_type="Signed  (2's comp)"  force_bin_pt=on  bin_pt=17  block_type=reinterpret
      settings: infoedit="Changes signal type without altering the binary representation.   You can changed the signal between signed and unsigned, and relocate the binary point.<br><br>Hardware notes: In hardware this block costs nothing.<br><br>Example:  Suppose the input is 6 bits wide, signed, with 2 fractional bits, and the output is forced to unsigned with 0 fractional bits.  Then an input of -2.0 (1110.00 in binary 2's complement) becomes an output of 56 (111000 in binary)."
  force_re : xbsIndex_r4/Reinterpret  force_arith_type=on  arith_type="Signed  (2's comp)"  force_bin_pt=on  bin_pt=17  block_type=reinterpret
      settings: infoedit="Changes signal type without altering the binary representation.   You can changed the signal between signed and unsigned, and relocate the binary point.<br><br>Hardware notes: In hardware this block costs nothing.<br><br>Example:  Suppose the input is 6 bits wide, signed, with 2 fractional bits, and the output is forced to unsigned with 0 fractional bits.  Then an input of -2.0 (1110.00 in binary 2's complement) becomes an output of 56 (111000 in binary)."
  slice_im : xbsIndex_r4/Slice  nbits=18  boolean_output=off  mode=Lower Bit Location + Width  bit1=0  base1=MSB of Input  bit0=0  base0=LSB of Input  dbl_ovrd=off  block_type=slice
  slice_re : xbsIndex_r4/Slice  nbits=18  boolean_output=off  mode=Upper Bit Location + Width  bit1=0  base1=MSB of Input  bit0=0  base0=LSB of Input  dbl_ovrd=off  block_type=slice
  re : Outport
  im : Outport  Port=2
  wires:
  c/1 -> slice_re/1, slice_im/1
  slice_re/1 -> force_re/1
  slice_im/1 -> force_im/1
  force_re/1 -> re/1
  force_im/1 -> im/1

== system fft_test_BG/fft_BG_xblock/fft_direct_xblock/butterfly2_0/twiddle/c_to_ri_b ==
  c : Inport
  force_im : xbsIndex_r4/Reinterpret  force_arith_type=on  arith_type="Signed  (2's comp)"  force_bin_pt=on  bin_pt=17  block_type=reinterpret
      settings: infoedit="Changes signal type without altering the binary representation.   You can changed the signal between signed and unsigned, and relocate the binary point.<br><br>Hardware notes: In hardware this block costs nothing.<br><br>Example:  Suppose the input is 6 bits wide, signed, with 2 fractional bits, and the output is forced to unsigned with 0 fractional bits.  Then an input of -2.0 (1110.00 in binary 2's complement) becomes an output of 56 (111000 in binary)."
  force_re : xbsIndex_r4/Reinterpret  force_arith_type=on  arith_type="Signed  (2's comp)"  force_bin_pt=on  bin_pt=17  block_type=reinterpret
      settings: infoedit="Changes signal type without altering the binary representation.   You can changed the signal between signed and unsigned, and relocate the binary point.<br><br>Hardware notes: In hardware this block costs nothing.<br><br>Example:  Suppose the input is 6 bits wide, signed, with 2 fractional bits, and the output is forced to unsigned with 0 fractional bits.  Then an input of -2.0 (1110.00 in binary 2's complement) becomes an output of 56 (111000 in binary)."
  slice_im : xbsIndex_r4/Slice  nbits=18  boolean_output=off  mode=Lower Bit Location + Width  bit1=0  base1=MSB of Input  bit0=0  base0=LSB of Input  dbl_ovrd=off  block_type=slice
  slice_re : xbsIndex_r4/Slice  nbits=18  boolean_output=off  mode=Upper Bit Location + Width  bit1=0  base1=MSB of Input  bit0=0  base0=LSB of Input  dbl_ovrd=off  block_type=slice
  re : Outport
  im : Outport  Port=2
  wires:
  c/1 -> slice_re/1, slice_im/1
  slice_re/1 -> force_re/1
  slice_im/1 -> force_im/1
  force_re/1 -> re/1
  force_im/1 -> im/1

== system fft_test_BG/fft_BG_xblock/fft_direct_xblock/butterfly2_0/twiddle/c_to_ri_w ==
  c : Inport
  force_im : xbsIndex_r4/Reinterpret  force_arith_type=on  arith_type="Signed  (2's comp)"  force_bin_pt=on  bin_pt=16  block_type=reinterpret
      settings: infoedit="Changes signal type without altering the binary representation.   You can changed the signal between signed and unsigned, and relocate the binary point.<br><br>Hardware notes: In hardware this block costs nothing.<br><br>Example:  Suppose the input is 6 bits wide, signed, with 2 fractional bits, and the output is forced to unsigned with 0 fractional bits.  Then an input of -2.0 (1110.00 in binary 2's complement) becomes an output of 56 (111000 in binary)."
  force_re : xbsIndex_r4/Reinterpret  force_arith_type=on  arith_type="Signed  (2's comp)"  force_bin_pt=on  bin_pt=16  block_type=reinterpret
      settings: infoedit="Changes signal type without altering the binary representation.   You can changed the signal between signed and unsigned, and relocate the binary point.<br><br>Hardware notes: In hardware this block costs nothing.<br><br>Example:  Suppose the input is 6 bits wide, signed, with 2 fractional bits, and the output is forced to unsigned with 0 fractional bits.  Then an input of -2.0 (1110.00 in binary 2's complement) becomes an output of 56 (111000 in binary)."
  slice_im : xbsIndex_r4/Slice  nbits=18  boolean_output=off  mode=Lower Bit Location + Width  bit1=0  base1=MSB of Input  bit0=0  base0=LSB of Input  dbl_ovrd=off  block_type=slice
  slice_re : xbsIndex_r4/Slice  nbits=18  boolean_output=off  mode=Upper Bit Location + Width  bit1=0  base1=MSB of Input  bit0=0  base0=LSB of Input  dbl_ovrd=off  block_type=slice
  re : Outport
  im : Outport  Port=2
  wires:
  c/1 -> slice_re/1, slice_im/1
  slice_re/1 -> force_re/1
  slice_im/1 -> force_im/1
  force_re/1 -> re/1
  force_im/1 -> im/1
